simulink library "lib_all"  (Simulink 7.6)
settings: Solver=ode45  SolverMode=SingleTasking  StartTime=0.0  StopTime=10.0  RelTol=1e-3
values from neighbouring files (symbols this model uses):
  map_lib_03 = [6.8679 7.0159 7.4571 8.1849 9.1892 10.456 11.97 13.71 15.655 17.781 20.063 22.474 ... (803 elements, 73x11)]    [scr_simdata.mat]    (also in scr_simdata_all.mat)
  map_lib_06 = [2.5784 2.6727 2.9588 3.4453 4.1444 5.068 6.226 7.6242 9.2634 11.139 13.24 15.552 ... (803 elements, 73x11)]    [scr_simdata.mat]    (also in scr_simdata_all.mat)
  map_lib_09 = [0.0025784 0.0026727 0.0029588 0.0034453 0.0041444 0.005068 0.006226 0.0076242 0.0092634 0.011139 0.01324 0.015552 ... (803 elements, 73x11)]    [scr_simdata.mat]    (also in scr_simdata_all.mat)
  map_lib_10 = [2.74 2.75 2.76 2.77 2.78 2.79 2.8 2.81 2.82 2.83 2.84 2.85 ... (81 elements, 1x81)]    [scr_simdata.mat]    (also in scr_simdata_all.mat)
  map_lib_11 = [1.1983 1.2027 1.207 1.2113 1.2156 1.2198 1.2241 1.2283 1.2326 1.2368 1.241 1.2451 ... (81 elements, 1x81)]    [scr_simdata.mat]    (also in scr_simdata_all.mat)
  map_lib_12 = [2.74 2.75 2.76 2.77 2.78 2.79 2.8 2.81 2.82 2.83 2.84 2.85 ... (81 elements, 1x81)]    [scr_simdata.mat]    (also in scr_simdata_all.mat)
  map_lib_13 = [1.74 1.75 1.76 1.77 1.78 1.79 1.8 1.81 1.82 1.83 1.84 1.85 ... (81 elements, 1x81)]    [scr_simdata.mat]    (also in scr_simdata_all.mat)
  map_lib_16 = [0.00048828125 1.6159 1.8962 2.0821 2.2251 2.3426 2.4433 2.5318 2.611 2.683 2.749 2.8101 ... (1419 elements, 129x11)]    [scr_simdata.mat]    (also in scr_simdata_all.mat)
  map_mr2_01 = [0 800 1600 2400 3200 4000]    [scr_simdata.mat]    (also in scr_simdata_all.mat)
  map_mr2_02 = [1.32 1.32 1.33 1.34 1.36 1.38]    [scr_simdata.mat]    (also in scr_simdata_all.mat)
  map_mr2_03 = [0 800 1600 2400 3200 4000]    [scr_simdata.mat]    (also in scr_simdata_all.mat)
  map_mr2_04 = [1.32 1.32 1.33 1.34 1.36 1.38]    [scr_simdata.mat]    (also in scr_simdata_all.mat)
  map_mr2_05 = [0 800 1600 2400 3200 4000]    [scr_simdata.mat]    (also in scr_simdata_all.mat)
  map_mr2_06 = [1.32 1.32 1.33 1.34 1.36 1.38]    [scr_simdata.mat]    (also in scr_simdata_all.mat)
  Parameter1 = 6    [scr_simdata.mat]    (also in scr_simdata_all.mat)
  Parameter101 = 30    [scr_simdata.mat]    (also in scr_simdata_all.mat)
  Parameter102 = 12    [scr_simdata.mat]    (also in scr_simdata_all.mat)
  Parameter103 = -240    [scr_simdata.mat]    (also in scr_simdata_all.mat)
  Parameter108 = 48    [scr_simdata.mat]    (also in scr_simdata_all.mat)
  Parameter120 = 16    [scr_simdata.mat]    (also in scr_simdata_all.mat)
  Parameter121 = 87    [scr_simdata.mat]    (also in scr_simdata_all.mat)
  Parameter123 = 78    [scr_simdata.mat]    (also in scr_simdata_all.mat)
  Parameter124 = 60    [scr_simdata.mat]    (also in scr_simdata_all.mat)
  Parameter125 = -480    [scr_simdata.mat]    (also in scr_simdata_all.mat)
  Parameter130 = 96    [scr_simdata.mat]    (also in scr_simdata_all.mat)
  Parameter131 = 3    [scr_simdata.mat]    (also in scr_simdata_all.mat)
  Parameter144 = 3    [scr_simdata.mat]    (also in scr_simdata_all.mat)
  Parameter18 = 128    [scr_simdata.mat]    (also in scr_simdata_all.mat)
  Parameter19 = 16    [scr_simdata.mat]    (also in scr_simdata_all.mat)
  Parameter2 = 12    [scr_simdata.mat]    (also in scr_simdata_all.mat)
  Parameter20 = 7    [scr_simdata.mat]    (also in scr_simdata_all.mat)
  Parameter21 = 4    [scr_simdata.mat]    (also in scr_simdata_all.mat)
  Parameter3 = 18    [scr_simdata.mat]    (also in scr_simdata_all.mat)
  Parameter32 = 1    [scr_simdata.mat]    (also in scr_simdata_all.mat)
  Parameter34 = 24    [scr_simdata.mat]    (also in scr_simdata_all.mat)
  Parameter4 = 138    [scr_simdata.mat]    (also in scr_simdata_all.mat)
  Parameter42 = 48    [scr_simdata.mat]    (also in scr_simdata_all.mat)
  Parameter5 = 135    [scr_simdata.mat]    (also in scr_simdata_all.mat)
  Parameter55 = 0    [scr_simdata.mat]    (also in scr_simdata_all.mat)
  Parameter56 = 0    [scr_simdata.mat]    (also in scr_simdata_all.mat)
  Parameter57 = 1    [scr_simdata.mat]    (also in scr_simdata_all.mat)
  Parameter58 = 1    [scr_simdata.mat]    (also in scr_simdata_all.mat)
  Parameter59 = 2    [scr_simdata.mat]    (also in scr_simdata_all.mat)
  Parameter6 = 132    [scr_simdata.mat]    (also in scr_simdata_all.mat)
  Parameter60 = 2    [scr_simdata.mat]    (also in scr_simdata_all.mat)
  Parameter63 = 144    [scr_simdata.mat]    (also in scr_simdata_all.mat)
  Parameter7 = 126    [scr_simdata.mat]    (also in scr_simdata_all.mat)
  Parameter76 = 64    [scr_simdata.mat]    (also in scr_simdata_all.mat)
  Parameter77 = 135    [scr_simdata.mat]    (also in scr_simdata_all.mat)
  Parameter79 = 126    [scr_simdata.mat]    (also in scr_simdata_all.mat)
  Parameter80 = 108    [scr_simdata.mat]    (also in scr_simdata_all.mat)
  Parameter81 = 0    [scr_simdata.mat]    (also in scr_simdata_all.mat)
  Parameter86 = 0    [scr_simdata.mat]    (also in scr_simdata_all.mat)
  Parameter98 = 112    [scr_simdata.mat]    (also in scr_simdata_all.mat)
  Parameter99 = 39    [scr_simdata.mat]    (also in scr_simdata_all.mat)
undefined symbols (not defined in the file or in its folder): cylvolkp_hih_map2h, cylvolkp_map2h, pk1k_map2h
libraries: simulink x2
(part 20 of 36)

== system lib_all/SubSystem176/SubSystem329/SubSystem332/SubSystem337/SubSystem340 ==
  note: ***編集禁止***
  element : Inport
  Inside_DSR : DataStoreRead  DataStoreName=DSM379
  Selector : Selector  IndexOptions=Index vector (port)  Indices=[1 3]  OutputSizes=1
  Output : Outport
  wires:
  Selector/1 -> Output/1
  element/1 -> Selector/2
  Inside_DSR/1 -> Selector/1

== system lib_all/SubSystem176/SubSystem329/SubSystem332/SubSystem341/SubSystem342 ==
  note: ***編集禁止***
  input : Inport
  element : Inport  Port=2
  Assignment : Assignment  IndexOptions=Index vector (port)  Indices=1  OutputSizes=[1 1]
  Inside_DSR : DataStoreRead  DataStoreName=DSM378
  Inside_DSW : DataStoreWrite  DataStoreName=DSM378
  wires:
  element/1 -> Assignment/3
  input/1 -> Assignment/2
  Inside_DSR/1 -> Assignment/1
  Assignment/1 -> Inside_DSW/1

== system lib_all/SubSystem1770/SubSystem1774/SubSystem1778/SubSystem1779/SingleForIterator ==
  note: ***編集禁止***
  N : Inport
  For_loop : Stateflow chart  TreatAsAtomicUnit=on
  action : Outport
  i : Outport  Port=2
  wires:
  For_loop/2(function_call) -> action/1
  N/1 -> For_loop/1(N_Loop)
  For_loop/1(idx) -> i/1

== system lib_all/SubSystem1770/SubSystem1774/SubSystem1778/SubSystem1779/SubSystem1783 ==
  note: Text348
  Block4866 : Inport
  Block4867 : Inport  Port=2
  Block4868 : Inport  Port=3
  Block4869 : TriggerPort  TriggerType=function-call
  Block4870 : Constant  Value=0  OutDataTypeStr=double  SampleTime=-1
  Block4871 : Constant  Value=3  OutDataTypeStr=double  SampleTime=-1
  Block4872 : Constant  OutDataTypeStr=double  SampleTime=-1
  Block4873 : Constant  Value=Parameter63  OutDataTypeStr=double  SampleTime=-1
  Block4874 : Constant  OutDataTypeStr=double  SampleTime=-1
  Block4875 : Constant  OutDataTypeStr=double  SampleTime=-1
  Block4876 : Sum  Inputs=+-  OutDataTypeStr=double  RndMeth=Zero  SaturateOnIntegerOverflow=on  InputSameDT=on
  Block{4877..4881} (5 alike) : Sum  OutDataTypeStr=double  RndMeth=Zero  SaturateOnIntegerOverflow=on  InputSameDT=on
  Block4882 : Switch  Threshold=0.5  RndMeth=Zero  SaturateOnIntegerOverflow=on  InputSameDT=on
  Block4883 : Switch  Threshold=0.5  RndMeth=Zero  SaturateOnIntegerOverflow=on  InputSameDT=on
  Block4884 : UnitDelay
  SubSystem1784 : Subsystem
  SubSystem1785 : Subsystem
  SubSystem1786 : Subsystem
  Block4889 : Outport
  wires:
  Block4879/1 -> Block4882/3
  Block4884/1 -> Block4879/2
  SubSystem1786/1(Block4888) -> Block4879/1, Block4882/1
  Block4882/1 -> Block4884/1, Block4889/1
  Block4878/1 -> Block4883/1
  Block4873/1 -> Block4878/1
  SubSystem1784/1(Out1) -> Block4883/2
  SubSystem1785/1(Out1) -> Block4882/2
  Block4872/1 -> SubSystem1785/1(In1)
  Block4870/1 -> SubSystem1784/2(In2)
  Block4877/1 -> SubSystem1784/1(In1), Block4878/2, Block4883/3
  Block4880/1 -> SubSystem1786/1(Block4885)
  Block4867/1 -> Block4880/1
  Block4868/1 -> Block4877/2
  Block4876/1 -> Block4877/1
  Block4871/1 -> Block4876/2
  Block4866/1 -> SubSystem1785/2(In2), Block4876/1
  Block4874/1 -> Block4880/2
  Block4883/1 -> Block4881/1
  Block4875/1 -> Block4881/2
  Block4881/1 -> SubSystem1786/2(Block4886)

== system lib_all/SubSystem1770/SubSystem1774/SubSystem1791/SubSystem1792/SubSystem1794 ==
  note: ***編集禁止***
  N : Inport
  For_loop : Stateflow chart  TreatAsAtomicUnit=on
  action : Outport
  i : Outport  Port=2
  wires:
  For_loop/2(function_call) -> action/1
  N/1 -> For_loop/1(N_Loop)
  For_loop/1(idx) -> i/1

== system lib_all/SubSystem1770/SubSystem1774/SubSystem1791/SubSystem1792/SubSystem1796 ==
  note: Text351
  Block4905 : Inport
  Block4906 : Inport  Port=2
  Block4907 : Inport  Port=3
  Block4908 : TriggerPort  TriggerType=function-call
  Block4909 : Constant  Value=0  OutDataTypeStr=double  SampleTime=-1
  Block4910 : Constant  Value=3  OutDataTypeStr=double  SampleTime=-1
  Block4911 : Constant  OutDataTypeStr=double  SampleTime=-1
  Block4912 : Constant  Value=Parameter63  OutDataTypeStr=double  SampleTime=-1
  Block4913 : Constant  OutDataTypeStr=double  SampleTime=-1
  Block4914 : Constant  OutDataTypeStr=double  SampleTime=-1
  Block4915 : Sum  Inputs=+-  OutDataTypeStr=double  RndMeth=Zero  SaturateOnIntegerOverflow=on  InputSameDT=on
  Block{4916..4920} (5 alike) : Sum  OutDataTypeStr=double  RndMeth=Zero  SaturateOnIntegerOverflow=on  InputSameDT=on
  Block4921 : Switch  Threshold=0.5  RndMeth=Zero  SaturateOnIntegerOverflow=on  InputSameDT=on
  Block4922 : Switch  Threshold=0.5  RndMeth=Zero  SaturateOnIntegerOverflow=on  InputSameDT=on
  Block4923 : UnitDelay
  SubSystem1797 : Subsystem
  SubSystem1798 : Subsystem
  SubSystem1799 : Subsystem
  Block4928 : Outport
  wires:
  Block4918/1 -> Block4921/3
  Block4923/1 -> Block4918/2
  SubSystem1799/1(Block4927) -> Block4918/1, Block4921/1
  Block4921/1 -> Block4923/1, Block4928/1
  Block4917/1 -> Block4922/1
  Block4912/1 -> Block4917/1
  SubSystem1797/1(Out1) -> Block4922/2
  SubSystem1798/1(Out1) -> Block4921/2
  Block4911/1 -> SubSystem1798/1(In1)
  Block4909/1 -> SubSystem1797/2(In2)
  Block4916/1 -> SubSystem1797/1(In1), Block4917/2, Block4922/3
  Block4919/1 -> SubSystem1799/1(Block4924)
  Block4906/1 -> Block4919/1
  Block4907/1 -> Block4916/2
  Block4915/1 -> Block4916/1
  Block4910/1 -> Block4915/2
  Block4905/1 -> SubSystem1798/2(In2), Block4915/1
  Block4913/1 -> Block4919/2
  Block4922/1 -> Block4920/1
  Block4914/1 -> Block4920/2
  Block4920/1 -> SubSystem1799/2(Block4925)

== system lib_all/SubSystem1770/SubSystem1774/SubSystem1804/SubSystem1805/SubSystem1807 ==
  note: ***編集禁止***
  N : Inport
  For_loop : Stateflow chart  TreatAsAtomicUnit=on
  action : Outport
  i : Outport  Port=2
  wires:
  For_loop/2(function_call) -> action/1
  N/1 -> For_loop/1(N_Loop)
  For_loop/1(idx) -> i/1

== system lib_all/SubSystem1770/SubSystem1774/SubSystem1804/SubSystem1805/SubSystem1809 ==
  note: Text354
  Block4944 : Inport
  Block4945 : Inport  Port=2
  Block4946 : Inport  Port=3
  Block4947 : TriggerPort  TriggerType=function-call
  Block4948 : Constant  Value=0  OutDataTypeStr=double  SampleTime=-1
  Block4949 : Constant  Value=3  OutDataTypeStr=double  SampleTime=-1
  Block4950 : Constant  OutDataTypeStr=double  SampleTime=-1
  Block4951 : Constant  Value=Parameter63  OutDataTypeStr=double  SampleTime=-1
  Block4952 : Constant  OutDataTypeStr=double  SampleTime=-1
  Block4953 : Constant  OutDataTypeStr=double  SampleTime=-1
  Block4954 : Sum  Inputs=+-  OutDataTypeStr=double  RndMeth=Zero  SaturateOnIntegerOverflow=on  InputSameDT=on
  Block{4955..4959} (5 alike) : Sum  OutDataTypeStr=double  RndMeth=Zero  SaturateOnIntegerOverflow=on  InputSameDT=on
  Block4960 : Switch  Threshold=0.5  RndMeth=Zero  SaturateOnIntegerOverflow=on  InputSameDT=on
  Block4961 : Switch  Threshold=0.5  RndMeth=Zero  SaturateOnIntegerOverflow=on  InputSameDT=on
  Block4962 : UnitDelay
  SubSystem1810 : Subsystem
  SubSystem1811 : Subsystem
  SubSystem1812 : Subsystem
  Block4967 : Outport
  wires:
  Block4957/1 -> Block4960/3
  Block4962/1 -> Block4957/2
  SubSystem1812/1(Block4966) -> Block4957/1, Block4960/1
  Block4960/1 -> Block4962/1, Block4967/1
  Block4956/1 -> Block4961/1
  Block4951/1 -> Block4956/1
  SubSystem1810/1(Out1) -> Block4961/2
  SubSystem1811/1(Out1) -> Block4960/2
  Block4950/1 -> SubSystem1811/1(In1)
  Block4948/1 -> SubSystem1810/2(In2)
  Block4955/1 -> SubSystem1810/1(In1), Block4956/2, Block4961/3
  Block4958/1 -> SubSystem1812/1(Block4963)
  Block4945/1 -> Block4958/1
  Block4946/1 -> Block4955/2
  Block4954/1 -> Block4955/1
  Block4949/1 -> Block4954/2
  Block4944/1 -> SubSystem1811/2(In2), Block4954/1
  Block4952/1 -> Block4958/2
  Block4961/1 -> Block4959/1
  Block4953/1 -> Block4959/2
  Block4959/1 -> SubSystem1812/2(Block4964)

== system lib_all/SubSystem1770/SubSystem1817/SubSystem1821/SubSystem1822/SubSystem1824 ==
  note: ***編集禁止***
  N : Inport
  For_loop : Stateflow chart  TreatAsAtomicUnit=on
  action : Outport
  i : Outport  Port=2
  wires:
  For_loop/2(function_call) -> action/1
  N/1 -> For_loop/1(N_Loop)
  For_loop/1(idx) -> i/1

== system lib_all/SubSystem1770/SubSystem1817/SubSystem1821/SubSystem1822/SubSystem1826 ==
  note: Text357
  Block4992 : Inport
  Block4993 : Inport  Port=2
  Block4994 : Inport  Port=3
  Block4995 : TriggerPort  TriggerType=function-call
  Block4996 : Constant  Value=0  OutDataTypeStr=double  SampleTime=-1
  Block4997 : Constant  Value=3  OutDataTypeStr=double  SampleTime=-1
  Block4998 : Constant  OutDataTypeStr=double  SampleTime=-1
  Block4999 : Constant  Value=Parameter63  OutDataTypeStr=double  SampleTime=-1
  Block5000 : Constant  OutDataTypeStr=double  SampleTime=-1
  Block5001 : Constant  OutDataTypeStr=double  SampleTime=-1
  Block5002 : Sum  Inputs=+-  OutDataTypeStr=double  RndMeth=Zero  SaturateOnIntegerOverflow=on  InputSameDT=on
  Block{5003..5007} (5 alike) : Sum  OutDataTypeStr=double  RndMeth=Zero  SaturateOnIntegerOverflow=on  InputSameDT=on
  Block5008 : Switch  Threshold=0.5  RndMeth=Zero  SaturateOnIntegerOverflow=on  InputSameDT=on
  Block5009 : Switch  Threshold=0.5  RndMeth=Zero  SaturateOnIntegerOverflow=on  InputSameDT=on
  Block5010 : UnitDelay
  SubSystem1827 : Subsystem
  SubSystem1828 : Subsystem
  SubSystem1829 : Subsystem
  Block5015 : Outport
  wires:
  Block5005/1 -> Block5008/3
  Block5010/1 -> Block5005/2
  SubSystem1829/1(Block5014) -> Block5005/1, Block5008/1
  Block5008/1 -> Block5010/1, Block5015/1
  Block5004/1 -> Block5009/1
  Block4999/1 -> Block5004/1
  SubSystem1827/1(Out1) -> Block5009/2
  SubSystem1828/1(Out1) -> Block5008/2
  Block4998/1 -> SubSystem1828/1(In1)
  Block4996/1 -> SubSystem1827/2(In2)
  Block5003/1 -> SubSystem1827/1(In1), Block5004/2, Block5009/3
  Block5006/1 -> SubSystem1829/1(Block5011)
  Block4993/1 -> Block5006/1
  Block4994/1 -> Block5003/2
  Block5002/1 -> Block5003/1
  Block4997/1 -> Block5002/2
  Block4992/1 -> SubSystem1828/2(In2), Block5002/1
  Block5000/1 -> Block5006/2
  Block5009/1 -> Block5007/1
  Block5001/1 -> Block5007/2
  Block5007/1 -> SubSystem1829/2(Block5012)

== system lib_all/SubSystem1770/SubSystem1817/SubSystem1834/SubSystem1835/SubSystem1837 ==
  note: ***編集禁止***
  N : Inport
  For_loop : Stateflow chart  TreatAsAtomicUnit=on
  action : Outport
  i : Outport  Port=2
  wires:
  For_loop/2(function_call) -> action/1
  N/1 -> For_loop/1(N_Loop)
  For_loop/1(idx) -> i/1

== system lib_all/SubSystem1770/SubSystem1817/SubSystem1834/SubSystem1835/SubSystem1839 ==
  note: Text360
  Block5031 : Inport
  Block5032 : Inport  Port=2
  Block5033 : Inport  Port=3
  Block5034 : TriggerPort  TriggerType=function-call
  Block5035 : Constant  Value=0  OutDataTypeStr=double  SampleTime=-1
  Block5036 : Constant  Value=3  OutDataTypeStr=double  SampleTime=-1
  Block5037 : Constant  OutDataTypeStr=double  SampleTime=-1
  Block5038 : Constant  Value=Parameter63  OutDataTypeStr=double  SampleTime=-1
  Block5039 : Constant  OutDataTypeStr=double  SampleTime=-1
  Block5040 : Constant  OutDataTypeStr=double  SampleTime=-1
  Block5041 : Sum  Inputs=+-  OutDataTypeStr=double  RndMeth=Zero  SaturateOnIntegerOverflow=on  InputSameDT=on
  Block{5042..5046} (5 alike) : Sum  OutDataTypeStr=double  RndMeth=Zero  SaturateOnIntegerOverflow=on  InputSameDT=on
  Block5047 : Switch  Threshold=0.5  RndMeth=Zero  SaturateOnIntegerOverflow=on  InputSameDT=on
  Block5048 : Switch  Threshold=0.5  RndMeth=Zero  SaturateOnIntegerOverflow=on  InputSameDT=on
  Block5049 : UnitDelay
  SubSystem1840 : Subsystem
  SubSystem1841 : Subsystem
  SubSystem1842 : Subsystem
  Block5054 : Outport
  wires:
  Block5044/1 -> Block5047/3
  Block5049/1 -> Block5044/2
  SubSystem1842/1(Block5053) -> Block5044/1, Block5047/1
  Block5047/1 -> Block5049/1, Block5054/1
  Block5043/1 -> Block5048/1
  Block5038/1 -> Block5043/1
  SubSystem1840/1(Out1) -> Block5048/2
  SubSystem1841/1(Out1) -> Block5047/2
  Block5037/1 -> SubSystem1841/1(In1)
  Block5035/1 -> SubSystem1840/2(In2)
  Block5042/1 -> SubSystem1840/1(In1), Block5043/2, Block5048/3
  Block5045/1 -> SubSystem1842/1(Block5050)
  Block5032/1 -> Block5045/1
  Block5033/1 -> Block5042/2
  Block5041/1 -> Block5042/1
  Block5036/1 -> Block5041/2
  Block5031/1 -> SubSystem1841/2(In2), Block5041/1
  Block5039/1 -> Block5045/2
  Block5048/1 -> Block5046/1
  Block5040/1 -> Block5046/2
  Block5046/1 -> SubSystem1842/2(Block5051)

== system lib_all/SubSystem1770/SubSystem1817/SubSystem1847/SubSystem1848/SubSystem1850 ==
  note: ***編集禁止***
  N : Inport
  For_loop : Stateflow chart  TreatAsAtomicUnit=on
  action : Outport
  i : Outport  Port=2
  wires:
  For_loop/2(function_call) -> action/1
  N/1 -> For_loop/1(N_Loop)
  For_loop/1(idx) -> i/1

== system lib_all/SubSystem1770/SubSystem1817/SubSystem1847/SubSystem1848/SubSystem1852 ==
  note: Text363
  Block5070 : Inport
  Block5071 : Inport  Port=2
  Block5072 : Inport  Port=3
  Block5073 : TriggerPort  TriggerType=function-call
  Block5074 : Constant  Value=0  OutDataTypeStr=double  SampleTime=-1
  Block5075 : Constant  Value=3  OutDataTypeStr=double  SampleTime=-1
  Block5076 : Constant  OutDataTypeStr=double  SampleTime=-1
  Block5077 : Constant  Value=Parameter63  OutDataTypeStr=double  SampleTime=-1
  Block5078 : Constant  OutDataTypeStr=double  SampleTime=-1
  Block5079 : Constant  OutDataTypeStr=double  SampleTime=-1
  Block5080 : Sum  Inputs=+-  OutDataTypeStr=double  RndMeth=Zero  SaturateOnIntegerOverflow=on  InputSameDT=on
  Block{5081..5085} (5 alike) : Sum  OutDataTypeStr=double  RndMeth=Zero  SaturateOnIntegerOverflow=on  InputSameDT=on
  Block5086 : Switch  Threshold=0.5  RndMeth=Zero  SaturateOnIntegerOverflow=on  InputSameDT=on
  Block5087 : Switch  Threshold=0.5  RndMeth=Zero  SaturateOnIntegerOverflow=on  InputSameDT=on
  Block5088 : UnitDelay
  SubSystem1853 : Subsystem
  SubSystem1854 : Subsystem
  SubSystem1855 : Subsystem
  Block5093 : Outport
  wires:
  Block5083/1 -> Block5086/3
  Block5088/1 -> Block5083/2
  SubSystem1855/1(Block5092) -> Block5083/1, Block5086/1
  Block5086/1 -> Block5088/1, Block5093/1
  Block5082/1 -> Block5087/1
  Block5077/1 -> Block5082/1
  SubSystem1853/1(Out1) -> Block5087/2
  SubSystem1854/1(Out1) -> Block5086/2
  Block5076/1 -> SubSystem1854/1(In1)
  Block5074/1 -> SubSystem1853/2(In2)
  Block5081/1 -> SubSystem1853/1(In1), Block5082/2, Block5087/3
  Block5084/1 -> SubSystem1855/1(Block5089)
  Block5071/1 -> Block5084/1
  Block5072/1 -> Block5081/2
  Block5080/1 -> Block5081/1
  Block5075/1 -> Block5080/2
  Block5070/1 -> SubSystem1854/2(In2), Block5080/1
  Block5078/1 -> Block5084/2
  Block5087/1 -> Block5085/1
  Block5079/1 -> Block5085/2
  Block5085/1 -> SubSystem1855/2(Block5090)

== system lib_all/SubSystem1879/SubSystem1887/SubSystem1891/SubSystem1892/SubSystem1893 ==
  note: ***編集禁止***
  element : Inport
  Inside_DSR : DataStoreRead  DataStoreName=DSM18
  Selector : Selector  IndexOptions=Index vector (port)  Indices=[1 3]  OutputSizes=1
  Output : Outport
  wires:
  Inside_DSR/1 -> Selector/1
  element/1 -> Selector/2
  Selector/1 -> Output/1

== system lib_all/SubSystem1879/SubSystem1887/SubSystem1891/SubSystem1892/SubSystem1894 ==
  note: ***編集禁止***
  element : Inport
  Inside_DSR : DataStoreRead  DataStoreName=DSM8
  Selector : Selector  IndexOptions=Index vector (port)  Indices=[1 3]  OutputSizes=1
  Output : Outport
  wires:
  Selector/1 -> Output/1
  element/1 -> Selector/2
  Inside_DSR/1 -> Selector/1

== system lib_all/SubSystem1879/SubSystem1887/SubSystem1891/SubSystem1892/SubSystem1895 ==
  note: ***編集禁止***
  element : Inport
  Inside_DSR : DataStoreRead  DataStoreName=DSM11
  Selector : Selector  IndexOptions=Index vector (port)  Indices=[1 3]  OutputSizes=1
  Output : Outport
  wires:
  Inside_DSR/1 -> Selector/1
  element/1 -> Selector/2
  Selector/1 -> Output/1

== system lib_all/SubSystem1879/SubSystem1887/SubSystem1891/SubSystem1896/SubSystem1897 ==
  note: ***編集禁止***
  element : Inport
  Inside_DSR : DataStoreRead  DataStoreName=DSM18
  Selector : Selector  IndexOptions=Index vector (port)  Indices=[1 3]  OutputSizes=1
  Output : Outport
  wires:
  Inside_DSR/1 -> Selector/1
  element/1 -> Selector/2
  Selector/1 -> Output/1

== system lib_all/SubSystem1879/SubSystem1887/SubSystem1891/SubSystem1896/SubSystem1898 ==
  note: ***編集禁止***
  element : Inport
  Inside_DSR : DataStoreRead  DataStoreName=DSM8
  Selector : Selector  IndexOptions=Index vector (port)  Indices=[1 3]  OutputSizes=1
  Output : Outport
  wires:
  Selector/1 -> Output/1
  element/1 -> Selector/2
  Inside_DSR/1 -> Selector/1

== system lib_all/SubSystem1879/SubSystem1887/SubSystem1891/SubSystem1896/SubSystem1899 ==
  note: ***編集禁止***
  element : Inport
  Inside_DSR : DataStoreRead  DataStoreName=DSM11
  Selector : Selector  IndexOptions=Index vector (port)  Indices=[1 3]  OutputSizes=1
  Output : Outport
  wires:
  Inside_DSR/1 -> Selector/1
  element/1 -> Selector/2
  Selector/1 -> Output/1

== system lib_all/SubSystem1879/SubSystem1887/SubSystem1900/SubSystem1902/SubSystem1903 ==
  note: ***編集禁止***
  element : Inport
  Inside_DSR : DataStoreRead  DataStoreName=DSM18
  Selector : Selector  IndexOptions=Index vector (port)  Indices=[1 3]  OutputSizes=1
  Output : Outport
  wires:
  Inside_DSR/1 -> Selector/1
  element/1 -> Selector/2
  Selector/1 -> Output/1

== system lib_all/SubSystem1879/SubSystem1887/SubSystem1900/SubSystem1902/SubSystem1904 ==
  note: ***編集禁止***
  element : Inport
  Inside_DSR : DataStoreRead  DataStoreName=DSM8
  Selector : Selector  IndexOptions=Index vector (port)  Indices=[1 3]  OutputSizes=1
  Output : Outport
  wires:
  Selector/1 -> Output/1
  element/1 -> Selector/2
  Inside_DSR/1 -> Selector/1

== system lib_all/SubSystem1879/SubSystem1887/SubSystem1900/SubSystem1902/SubSystem1905 ==
  note: ***編集禁止***
  element : Inport
  Inside_DSR : DataStoreRead  DataStoreName=DSM11
  Selector : Selector  IndexOptions=Index vector (port)  Indices=[1 3]  OutputSizes=1
  Output : Outport
  wires:
  Inside_DSR/1 -> Selector/1
  element/1 -> Selector/2
  Selector/1 -> Output/1

== system lib_all/SubSystem1879/SubSystem1887/SubSystem1900/SubSystem1906/SubSystem1907 ==
  note: ***編集禁止***
  element : Inport
  Inside_DSR : DataStoreRead  DataStoreName=DSM18
  Selector : Selector  IndexOptions=Index vector (port)  Indices=[1 3]  OutputSizes=1
  Output : Outport
  wires:
  Inside_DSR/1 -> Selector/1
  element/1 -> Selector/2
  Selector/1 -> Output/1

== system lib_all/SubSystem1879/SubSystem1887/SubSystem1900/SubSystem1906/SubSystem1908 ==
  note: ***編集禁止***
  element : Inport
  Inside_DSR : DataStoreRead  DataStoreName=DSM8
  Selector : Selector  IndexOptions=Index vector (port)  Indices=[1 3]  OutputSizes=1
  Output : Outport
  wires:
  Selector/1 -> Output/1
  element/1 -> Selector/2
  Inside_DSR/1 -> Selector/1

== system lib_all/SubSystem1879/SubSystem1887/SubSystem1900/SubSystem1906/SubSystem1909 ==
  note: ***編集禁止***
  element : Inport
  Inside_DSR : DataStoreRead  DataStoreName=DSM11
  Selector : Selector  IndexOptions=Index vector (port)  Indices=[1 3]  OutputSizes=1
  Output : Outport
  wires:
  Inside_DSR/1 -> Selector/1
  element/1 -> Selector/2
  Selector/1 -> Output/1

== system lib_all/SubSystem1879/SubSystem1887/SubSystem1910/SubSystem1912/SubSystem1913 ==
  note: ***編集禁止***
  element : Inport
  Inside_DSR : DataStoreRead  DataStoreName=DSM18
  Selector : Selector  IndexOptions=Index vector (port)  Indices=[1 3]  OutputSizes=1
  Output : Outport
  wires:
  Inside_DSR/1 -> Selector/1
  element/1 -> Selector/2
  Selector/1 -> Output/1

== system lib_all/SubSystem1879/SubSystem1887/SubSystem1910/SubSystem1912/SubSystem1914 ==
  note: ***編集禁止***
  element : Inport
  Inside_DSR : DataStoreRead  DataStoreName=DSM8
  Selector : Selector  IndexOptions=Index vector (port)  Indices=[1 3]  OutputSizes=1
  Output : Outport
  wires:
  Selector/1 -> Output/1
  element/1 -> Selector/2
  Inside_DSR/1 -> Selector/1

== system lib_all/SubSystem1879/SubSystem1887/SubSystem1910/SubSystem1912/SubSystem1915 ==
  note: ***編集禁止***
  element : Inport
  Inside_DSR : DataStoreRead  DataStoreName=DSM11
  Selector : Selector  IndexOptions=Index vector (port)  Indices=[1 3]  OutputSizes=1
  Output : Outport
  wires:
  Inside_DSR/1 -> Selector/1
  element/1 -> Selector/2
  Selector/1 -> Output/1

== system lib_all/SubSystem1879/SubSystem1887/SubSystem1910/SubSystem1916/SubSystem1917 ==
  note: ***編集禁止***
  element : Inport
  Inside_DSR : DataStoreRead  DataStoreName=DSM18
  Selector : Selector  IndexOptions=Index vector (port)  Indices=[1 3]  OutputSizes=1
  Output : Outport
  wires:
  Inside_DSR/1 -> Selector/1
  element/1 -> Selector/2
  Selector/1 -> Output/1

== system lib_all/SubSystem1879/SubSystem1887/SubSystem1910/SubSystem1916/SubSystem1918 ==
  note: ***編集禁止***
  element : Inport
  Inside_DSR : DataStoreRead  DataStoreName=DSM8
  Selector : Selector  IndexOptions=Index vector (port)  Indices=[1 3]  OutputSizes=1
  Output : Outport
  wires:
  Selector/1 -> Output/1
  element/1 -> Selector/2
  Inside_DSR/1 -> Selector/1

== system lib_all/SubSystem1879/SubSystem1887/SubSystem1910/SubSystem1916/SubSystem1919 ==
  note: ***編集禁止***
  element : Inport
  Inside_DSR : DataStoreRead  DataStoreName=DSM11
  Selector : Selector  IndexOptions=Index vector (port)  Indices=[1 3]  OutputSizes=1
  Output : Outport
  wires:
  Inside_DSR/1 -> Selector/1
  element/1 -> Selector/2
  Selector/1 -> Output/1

== system lib_all/SubSystem1920/SubSystem1928/SubSystem1932/SubSystem1933/SubSystem1934 ==
  note: ***編集禁止***
  element : Inport
  Inside_DSR : DataStoreRead  DataStoreName=DSM18
  Selector : Selector  IndexOptions=Index vector (port)  Indices=[1 3]  OutputSizes=1
  Output : Outport
  wires:
  Inside_DSR/1 -> Selector/1
  element/1 -> Selector/2
  Selector/1 -> Output/1

== system lib_all/SubSystem1920/SubSystem1928/SubSystem1932/SubSystem1933/SubSystem1935 ==
  note: ***編集禁止***
  element : Inport
  Inside_DSR : DataStoreRead  DataStoreName=DSM8
  Selector : Selector  IndexOptions=Index vector (port)  Indices=[1 3]  OutputSizes=1
  Output : Outport
  wires:
  Selector/1 -> Output/1
  element/1 -> Selector/2
  Inside_DSR/1 -> Selector/1

== system lib_all/SubSystem1920/SubSystem1928/SubSystem1932/SubSystem1933/SubSystem1936 ==
  note: ***編集禁止***
  element : Inport
  Inside_DSR : DataStoreRead  DataStoreName=DSM11
  Selector : Selector  IndexOptions=Index vector (port)  Indices=[1 3]  OutputSizes=1
  Output : Outport
  wires:
  Inside_DSR/1 -> Selector/1
  element/1 -> Selector/2
  Selector/1 -> Output/1

== system lib_all/SubSystem1920/SubSystem1928/SubSystem1932/SubSystem1937/SubSystem1938 ==
  note: ***編集禁止***
  element : Inport
  Inside_DSR : DataStoreRead  DataStoreName=DSM18
  Selector : Selector  IndexOptions=Index vector (port)  Indices=[1 3]  OutputSizes=1
  Output : Outport
  wires:
  Inside_DSR/1 -> Selector/1
  element/1 -> Selector/2
  Selector/1 -> Output/1

== system lib_all/SubSystem1920/SubSystem1928/SubSystem1932/SubSystem1937/SubSystem1939 ==
  note: ***編集禁止***
  element : Inport
  Inside_DSR : DataStoreRead  DataStoreName=DSM8
  Selector : Selector  IndexOptions=Index vector (port)  Indices=[1 3]  OutputSizes=1
  Output : Outport
  wires:
  Selector/1 -> Output/1
  element/1 -> Selector/2
  Inside_DSR/1 -> Selector/1

== system lib_all/SubSystem1920/SubSystem1928/SubSystem1932/SubSystem1937/SubSystem1940 ==
  note: ***編集禁止***
  element : Inport
  Inside_DSR : DataStoreRead  DataStoreName=DSM11
  Selector : Selector  IndexOptions=Index vector (port)  Indices=[1 3]  OutputSizes=1
  Output : Outport
  wires:
  Inside_DSR/1 -> Selector/1
  element/1 -> Selector/2
  Selector/1 -> Output/1

== system lib_all/SubSystem1920/SubSystem1928/SubSystem1941/SubSystem1943/SubSystem1944 ==
  note: ***編集禁止***
  element : Inport
  Inside_DSR : DataStoreRead  DataStoreName=DSM18
  Selector : Selector  IndexOptions=Index vector (port)  Indices=[1 3]  OutputSizes=1
  Output : Outport
  wires:
  Inside_DSR/1 -> Selector/1
  element/1 -> Selector/2
  Selector/1 -> Output/1

== system lib_all/SubSystem1920/SubSystem1928/SubSystem1941/SubSystem1943/SubSystem1945 ==
  note: ***編集禁止***
  element : Inport
  Inside_DSR : DataStoreRead  DataStoreName=DSM8
  Selector : Selector  IndexOptions=Index vector (port)  Indices=[1 3]  OutputSizes=1
  Output : Outport
  wires:
  Selector/1 -> Output/1
  element/1 -> Selector/2
  Inside_DSR/1 -> Selector/1

== system lib_all/SubSystem1920/SubSystem1928/SubSystem1941/SubSystem1943/SubSystem1946 ==
  note: ***編集禁止***
  element : Inport
  Inside_DSR : DataStoreRead  DataStoreName=DSM11
  Selector : Selector  IndexOptions=Index vector (port)  Indices=[1 3]  OutputSizes=1
  Output : Outport
  wires:
  Inside_DSR/1 -> Selector/1
  element/1 -> Selector/2
  Selector/1 -> Output/1

== system lib_all/SubSystem1920/SubSystem1928/SubSystem1941/SubSystem1947/SubSystem1948 ==
  note: ***編集禁止***
  element : Inport
  Inside_DSR : DataStoreRead  DataStoreName=DSM18
  Selector : Selector  IndexOptions=Index vector (port)  Indices=[1 3]  OutputSizes=1
  Output : Outport
  wires:
  Inside_DSR/1 -> Selector/1
  element/1 -> Selector/2
  Selector/1 -> Output/1

== system lib_all/SubSystem1920/SubSystem1928/SubSystem1941/SubSystem1947/SubSystem1949 ==
  note: ***編集禁止***
  element : Inport
  Inside_DSR : DataStoreRead  DataStoreName=DSM8
  Selector : Selector  IndexOptions=Index vector (port)  Indices=[1 3]  OutputSizes=1
  Output : Outport
  wires:
  Selector/1 -> Output/1
  element/1 -> Selector/2
  Inside_DSR/1 -> Selector/1

== system lib_all/SubSystem1920/SubSystem1928/SubSystem1941/SubSystem1947/SubSystem1950 ==
  note: ***編集禁止***
  element : Inport
  Inside_DSR : DataStoreRead  DataStoreName=DSM11
  Selector : Selector  IndexOptions=Index vector (port)  Indices=[1 3]  OutputSizes=1
  Output : Outport
  wires:
  Inside_DSR/1 -> Selector/1
  element/1 -> Selector/2
  Selector/1 -> Output/1

== system lib_all/SubSystem1920/SubSystem1928/SubSystem1951/SubSystem1953/SubSystem1954 ==
  note: ***編集禁止***
  element : Inport
  Inside_DSR : DataStoreRead  DataStoreName=DSM18
  Selector : Selector  IndexOptions=Index vector (port)  Indices=[1 3]  OutputSizes=1
  Output : Outport
  wires:
  Inside_DSR/1 -> Selector/1
  element/1 -> Selector/2
  Selector/1 -> Output/1

== system lib_all/SubSystem1920/SubSystem1928/SubSystem1951/SubSystem1953/SubSystem1955 ==
  note: ***編集禁止***
  element : Inport
  Inside_DSR : DataStoreRead  DataStoreName=DSM8
  Selector : Selector  IndexOptions=Index vector (port)  Indices=[1 3]  OutputSizes=1
  Output : Outport
  wires:
  Selector/1 -> Output/1
  element/1 -> Selector/2
  Inside_DSR/1 -> Selector/1

== system lib_all/SubSystem1920/SubSystem1928/SubSystem1951/SubSystem1953/SubSystem1956 ==
  note: ***編集禁止***
  element : Inport
  Inside_DSR : DataStoreRead  DataStoreName=DSM11
  Selector : Selector  IndexOptions=Index vector (port)  Indices=[1 3]  OutputSizes=1
  Output : Outport
  wires:
  Inside_DSR/1 -> Selector/1
  element/1 -> Selector/2
  Selector/1 -> Output/1

== system lib_all/SubSystem1920/SubSystem1928/SubSystem1951/SubSystem1957/SubSystem1958 ==
  note: ***編集禁止***
  element : Inport
  Inside_DSR : DataStoreRead  DataStoreName=DSM18
  Selector : Selector  IndexOptions=Index vector (port)  Indices=[1 3]  OutputSizes=1
  Output : Outport
  wires:
  Inside_DSR/1 -> Selector/1
  element/1 -> Selector/2
  Selector/1 -> Output/1

== system lib_all/SubSystem1920/SubSystem1928/SubSystem1951/SubSystem1957/SubSystem1959 ==
  note: ***編集禁止***
  element : Inport
  Inside_DSR : DataStoreRead  DataStoreName=DSM8
  Selector : Selector  IndexOptions=Index vector (port)  Indices=[1 3]  OutputSizes=1
  Output : Outport
  wires:
  Selector/1 -> Output/1
  element/1 -> Selector/2
  Inside_DSR/1 -> Selector/1

== system lib_all/SubSystem1920/SubSystem1928/SubSystem1951/SubSystem1957/SubSystem1960 ==
  note: ***編集禁止***
  element : Inport
  Inside_DSR : DataStoreRead  DataStoreName=DSM11
  Selector : Selector  IndexOptions=Index vector (port)  Indices=[1 3]  OutputSizes=1
  Output : Outport
  wires:
  Inside_DSR/1 -> Selector/1
  element/1 -> Selector/2
  Selector/1 -> Output/1

== system lib_all/SubSystem347/SubSystem349/SubSystem352/SubSystem353/SubSystem355 ==
  note: ***編集禁止***
  In1 : Inport
  In2 : Inport  Port=2
  LO1 : Logic
  RO1 : RelationalOperator  Operator=<  InputSameDT=on
  RO2 : RelationalOperator  Operator=~=  InputSameDT=on
  single1 : DataTypeConversion  OutDataTypeStr=single  SaturateOnIntegerOverflow=on  RndMeth=Zero
  single2 : DataTypeConversion  OutDataTypeStr=single  SaturateOnIntegerOverflow=on  RndMeth=Zero
  Out1 : Outport
  wires:
  In2/1 -> single2/1
  In1/1 -> single1/1
  single1/1 -> RO1/1, RO2/1
  single2/1 -> RO1/2, RO2/2
  RO1/1 -> LO1/1
  RO2/1 -> LO1/2
  LO1/1 -> Out1/1

== system lib_all/SubSystem347/SubSystem349/SubSystem352/SubSystem353/SubSystem356 ==
  note: Text54
  note: Text53
  Block789 : Inport
  Block790 : Inport  Port=2
  Block791 : Inport  Port=3
  Block792 : Constant  Value=720  OutDataTypeStr=double  SampleTime=-1
  Block793 : Constant  Value=0  OutDataTypeStr=double  SampleTime=-1
  Block794 : Constant  Value=0  OutDataTypeStr=double  SampleTime=-1
  Block795 : Constant  Value=5  OutDataTypeStr=double  SampleTime=-1
  Block796 : DataStoreRead  DataStoreName=DSM50
  Block797 : DataStoreRead  DataStoreName=DSM35
  Block798 : Gain  Gain=5  SaturateOnIntegerOverflow=on  OutDataTypeStr=Inherit: Same as input  ParamDataTypeStr=Inherit: Same as input
      settings: AttributesFormatString=Attribute180
  Block799 : Gain  Gain=5  SaturateOnIntegerOverflow=on  OutDataTypeStr=Inherit: Same as input  ParamDataTypeStr=Inherit: Same as input
      settings: AttributesFormatString=Attribute181
  Block800 : Gain  Gain=1/5  SaturateOnIntegerOverflow=on  OutDataTypeStr=Inherit: Same as input  ParamDataTypeStr=Inherit: Same as input
      settings: AttributesFormatString=Attribute182
  Block801 : Product  Inputs=*/  OutDataTypeStr=double  SaturateOnIntegerOverflow=on  RndMeth=Zero  InputSameDT=on
  Block802 : Rounding
  Block803 : Rounding
  Block804 : Sum  Inputs=+-+-  OutDataTypeStr=double  RndMeth=Zero  SaturateOnIntegerOverflow=on  InputSameDT=on
  Block805 : Sum  Inputs=-+  OutDataTypeStr=double  RndMeth=Zero  SaturateOnIntegerOverflow=on  InputSameDT=on
  SubSystem357 : Subsystem  TreatAsAtomicUnit=on  mask(***ATC.DIVISION***){bd_version=1.01.A, r0=チェック有り, r1=, r2=, r3=, r4=, r5=, r6=, r7=, r8=, r9=, c0=, c1=, c2=, c3=, c4=, c5=, c6=, c7=, c8=, c9=}
      mask prompts: bd_version "Version"
      mask help: これはAutoCからのブロックです．編集禁止．
      settings: OpenFcn=warndlg('変更禁止!');
      mask Help: web(ui_blockhelpmng(gcb));
  SubSystem358 : Subsystem  TreatAsAtomicUnit=on  mask(***ATC.DSRVEC***){bd_version=1.01.A, r0=DSM95, r1=-1, r2=, r3=, r4=, r5=, r6=, r7=, r8=, r9=, c0=, c1=, c2=, c3=, c4=, c5=, c6=, c7=, c8=, c9=}
      mask prompts: bd_version "Version"
      mask help: これはAutoCからのブロックです．編集禁止．
      settings: OpenFcn=warndlg('変更禁止!');
      mask Help: web(ui_blockhelpmng(gcb));
      mask Display: disp('ATC.DSRVEC')
  SubSystem359 : Subsystem
      settings: RTWSystemCode=Inline
  Block814 : Outport
  Block815 : Outport  Port=2
  wires:
  Block803/1 -> Block814/1
  Block800/1 -> Block803/1
  Block799/1 -> Block804/1
  Block796/1 -> Block804/2
  SubSystem358/1(Output) -> SubSystem357/1(mult)
  Block793/1 -> SubSystem357/3(init)
  SubSystem357/1(Out) -> Block804/3  "SigLabel18"
  Block789/1 -> Block799/1
  SubSystem359/1(Block813) -> Block800/1, Block805/2, Block801/1
  Block791/1 -> Block804/4
  Block792/1 -> SubSystem359/1(Block806)
  Block804/1 -> SubSystem359/2(Block807)  "SigLabel17"
  Block794/1 -> SubSystem359/3(Block808)
  Block790/1 -> SubSystem358/1(element)
  Block795/1 -> Block801/2
  Block801/1 -> Block802/1
  Block802/1 -> Block798/1
  Block798/1 -> Block805/1
  Block805/1 -> Block815/1
  Block797/1 -> SubSystem357/2(div)

== system lib_all/SubSystem347/SubSystem349/SubSystem352/SubSystem353/SubSystem362 ==
  note: Text57
  note: Text56
  note: Text55
  Block816 : Inport
  Block817 : Inport  Port=2
  Block818 : Inport  Port=3
  Block819 : Constant  Value=0  OutDataTypeStr=double  SampleTime=-1
  Block820 : Constant  OutDataTypeStr=double  SampleTime=-1
  Block821 : Constant  OutDataTypeStr=double  SampleTime=-1
  Block822 : Constant  Value=0  OutDataTypeStr=double  SampleTime=-1
  Block823 : Constant  Value=2  OutDataTypeStr=double  SampleTime=-1
  Block824 : Constant  OutDataTypeStr=double  SampleTime=-1
  Block825 : Constant  Value=143  OutDataTypeStr=double  SampleTime=-1
  Block826 : Demux  Outputs=2
  Block827 : Logic  Operator=NOT
  Block828 : Mux  Inputs=2
  Block829 : Mux  Inputs=2
  Block830 : Sum  OutDataTypeStr=double  RndMeth=Zero  SaturateOnIntegerOverflow=on  InputSameDT=on
  Block831 : Sum  OutDataTypeStr=double  RndMeth=Zero  SaturateOnIntegerOverflow=on  InputSameDT=on
  Block{832..835} (4 alike) : Switch  Threshold=0.5  RndMeth=Zero  SaturateOnIntegerOverflow=on  InputSameDT=on
  SubSystem363 : Subsystem
  SubSystem364 : Subsystem
  SubSystem365 : Subsystem
  SubSystem366 : Subsystem
  SubSystem367 : Subsystem  TreatAsAtomicUnit=on
  SubSystem376 : Subsystem  TreatAsAtomicUnit=on
  SubSystem386 : Subsystem  TreatAsAtomicUnit=on
  SubSystem396 : Subsystem  TreatAsAtomicUnit=on
  Block895 : Outport
  Block896 : Outport  Port=2
  wires:
  Block834/1 -> Block831/2
  Block824/1 -> Block831/1
  Block816/1 -> SubSystem363/1(In1), Block830/2, Block828/1
  Block825/1 -> SubSystem363/2(In2), Block829/1
  Block817/1 -> SubSystem364/1(In1), SubSystem365/1(In1), SubSystem366/1(In1)
  Block819/1 -> SubSystem364/2(In2)
  SubSystem364/1(Out1) -> Block832/2, SubSystem367/Enable
  SubSystem367/1(Block849) -> Block832/1
  Block820/1 -> SubSystem365/2(In2)
  SubSystem365/1(Out1) -> Block833/2, SubSystem376/Enable
  SubSystem376/1(Block864) -> Block833/1
  Block833/1 -> Block832/3
  Block831/1 -> SubSystem367/1(Block836), SubSystem376/1(Block850), SubSystem386/1(Block865), SubSystem396/1(Block880)
  Block826/1 -> Block895/1
  Block826/2 -> Block896/1
  Block832/1 -> Block826/1
  SubSystem363/1(Out1) -> Block834/2
  Block821/1 -> Block830/1
  Block822/1 -> Block829/2
  Block829/1 -> Block834/3
  Block830/1 -> Block828/2
  Block828/1 -> Block834/1
  Block823/1 -> SubSystem366/2(In2)
  SubSystem366/1(Out1) -> Block827/1, SubSystem386/Enable, Block835/2
  Block827/1 -> SubSystem396/Enable
  SubSystem386/1(Block879) -> Block835/1
  SubSystem396/1(Block894) -> Block835/3
  Block835/1 -> Block833/3
  Block818/1 -> SubSystem367/2(Block837), SubSystem376/2(Block851), SubSystem386/2(Block866), SubSystem396/2(Block881)

== system lib_all/SubSystem347/SubSystem349/SubSystem352/SubSystem406/SubSystem408 ==
  note: ***編集禁止***
  In1 : Inport
  In2 : Inport  Port=2
  LO1 : Logic
  RO1 : RelationalOperator  Operator=<  InputSameDT=on
  RO2 : RelationalOperator  Operator=~=  InputSameDT=on
  single1 : DataTypeConversion  OutDataTypeStr=single  SaturateOnIntegerOverflow=on  RndMeth=Zero
  single2 : DataTypeConversion  OutDataTypeStr=single  SaturateOnIntegerOverflow=on  RndMeth=Zero
  Out1 : Outport
  wires:
  In2/1 -> single2/1
  In1/1 -> single1/1
  single1/1 -> RO1/1, RO2/1
  single2/1 -> RO1/2, RO2/2
  RO1/1 -> LO1/1
  RO2/1 -> LO1/2
  LO1/1 -> Out1/1

== system lib_all/SubSystem347/SubSystem349/SubSystem352/SubSystem406/SubSystem409 ==
  note: Text60
  note: Text59
  Block911 : Inport
  Block912 : Inport  Port=2
  Block913 : Inport  Port=3
  Block914 : Constant  Value=720  OutDataTypeStr=double  SampleTime=-1
  Block915 : Constant  Value=0  OutDataTypeStr=double  SampleTime=-1
  Block916 : Constant  Value=0  OutDataTypeStr=double  SampleTime=-1
  Block917 : Constant  Value=5  OutDataTypeStr=double  SampleTime=-1
  Block918 : DataStoreRead  DataStoreName=DSM50
  Block919 : DataStoreRead  DataStoreName=DSM35
  Block920 : Gain  Gain=5  SaturateOnIntegerOverflow=on  OutDataTypeStr=Inherit: Same as input  ParamDataTypeStr=Inherit: Same as input
      settings: AttributesFormatString=Attribute199
  Block921 : Gain  Gain=5  SaturateOnIntegerOverflow=on  OutDataTypeStr=Inherit: Same as input  ParamDataTypeStr=Inherit: Same as input
      settings: AttributesFormatString=Attribute200
  Block922 : Gain  Gain=1/5  SaturateOnIntegerOverflow=on  OutDataTypeStr=Inherit: Same as input  ParamDataTypeStr=Inherit: Same as input
      settings: AttributesFormatString=Attribute201
  Block923 : Product  Inputs=*/  OutDataTypeStr=double  SaturateOnIntegerOverflow=on  RndMeth=Zero  InputSameDT=on
  Block924 : Rounding
  Block925 : Rounding
  Block926 : Sum  Inputs=+-+-  OutDataTypeStr=double  RndMeth=Zero  SaturateOnIntegerOverflow=on  InputSameDT=on
  Block927 : Sum  Inputs=-+  OutDataTypeStr=double  RndMeth=Zero  SaturateOnIntegerOverflow=on  InputSameDT=on
  SubSystem410 : Subsystem  TreatAsAtomicUnit=on  mask(***ATC.DIVISION***){bd_version=1.01.A, r0=チェック有り, r1=, r2=, r3=, r4=, r5=, r6=, r7=, r8=, r9=, c0=, c1=, c2=, c3=, c4=, c5=, c6=, c7=, c8=, c9=}
      mask prompts: bd_version "Version"
      mask help: これはAutoCからのブロックです．編集禁止．
      settings: OpenFcn=warndlg('変更禁止!');
      mask Help: web(ui_blockhelpmng(gcb));
  SubSystem411 : Subsystem  TreatAsAtomicUnit=on  mask(***ATC.DSRVEC***){bd_version=1.01.A, r0=DSM95, r1=-1, r2=, r3=, r4=, r5=, r6=, r7=, r8=, r9=, c0=, c1=, c2=, c3=, c4=, c5=, c6=, c7=, c8=, c9=}
      mask prompts: bd_version "Version"
      mask help: これはAutoCからのブロックです．編集禁止．
      settings: OpenFcn=warndlg('変更禁止!');
      mask Help: web(ui_blockhelpmng(gcb));
      mask Display: disp('ATC.DSRVEC')
  SubSystem412 : Subsystem
      settings: RTWSystemCode=Inline
  Block936 : Outport
  Block937 : Outport  Port=2
  wires:
  Block925/1 -> Block936/1
  Block922/1 -> Block925/1
  Block921/1 -> Block926/1
  Block918/1 -> Block926/2
  SubSystem411/1(Output) -> SubSystem410/1(mult)
  Block915/1 -> SubSystem410/3(init)
  SubSystem410/1(Out) -> Block926/3  "SigLabel21"
  Block911/1 -> Block921/1
  SubSystem412/1(Block935) -> Block922/1, Block927/2, Block923/1
  Block913/1 -> Block926/4
  Block914/1 -> SubSystem412/1(Block928)
  Block926/1 -> SubSystem412/2(Block929)  "SigLabel20"
  Block916/1 -> SubSystem412/3(Block930)
  Block912/1 -> SubSystem411/1(element)
  Block917/1 -> Block923/2
  Block923/1 -> Block924/1
  Block924/1 -> Block920/1
  Block920/1 -> Block927/1
  Block927/1 -> Block937/1
  Block919/1 -> SubSystem410/2(div)

== system lib_all/SubSystem347/SubSystem349/SubSystem352/SubSystem406/SubSystem415 ==
  note: Text63
  note: Text62
  note: Text61
  Block938 : Inport
  Block939 : Inport  Port=2
  Block940 : Inport  Port=3
  Block941 : Constant  Value=0  OutDataTypeStr=double  SampleTime=-1
  Block942 : Constant  OutDataTypeStr=double  SampleTime=-1
  Block943 : Constant  OutDataTypeStr=double  SampleTime=-1
  Block944 : Constant  Value=0  OutDataTypeStr=double  SampleTime=-1
  Block945 : Constant  Value=2  OutDataTypeStr=double  SampleTime=-1
  Block946 : Constant  OutDataTypeStr=double  SampleTime=-1
  Block947 : Constant  Value=143  OutDataTypeStr=double  SampleTime=-1
  Block948 : Demux  Outputs=2
  Block949 : Logic  Operator=NOT
  Block950 : Mux  Inputs=2
  Block951 : Mux  Inputs=2
  Block952 : Sum  OutDataTypeStr=double  RndMeth=Zero  SaturateOnIntegerOverflow=on  InputSameDT=on
  Block953 : Sum  OutDataTypeStr=double  RndMeth=Zero  SaturateOnIntegerOverflow=on  InputSameDT=on
  Block{954..957} (4 alike) : Switch  Threshold=0.5  RndMeth=Zero  SaturateOnIntegerOverflow=on  InputSameDT=on
  SubSystem416 : Subsystem
  SubSystem417 : Subsystem
  SubSystem418 : Subsystem
  SubSystem419 : Subsystem
  SubSystem420 : Subsystem  TreatAsAtomicUnit=on
  SubSystem429 : Subsystem  TreatAsAtomicUnit=on
  SubSystem439 : Subsystem  TreatAsAtomicUnit=on
  SubSystem449 : Subsystem  TreatAsAtomicUnit=on
  Block1017 : Outport
  Block1018 : Outport  Port=2
  wires:
  Block956/1 -> Block953/2
  Block946/1 -> Block953/1
  Block938/1 -> SubSystem416/1(In1), Block952/2, Block950/1
  Block947/1 -> SubSystem416/2(In2), Block951/1
  Block939/1 -> SubSystem417/1(In1), SubSystem418/1(In1), SubSystem419/1(In1)
  Block941/1 -> SubSystem417/2(In2)
  SubSystem417/1(Out1) -> Block954/2, SubSystem420/Enable
  SubSystem420/1(Block971) -> Block954/1
  Block942/1 -> SubSystem418/2(In2)
  SubSystem418/1(Out1) -> Block955/2, SubSystem429/Enable
  SubSystem429/1(Block986) -> Block955/1
  Block955/1 -> Block954/3
  Block953/1 -> SubSystem420/1(Block958), SubSystem429/1(Block972), SubSystem439/1(Block987), SubSystem449/1(Block1002)
  Block948/1 -> Block1017/1
  Block948/2 -> Block1018/1
  Block954/1 -> Block948/1
  SubSystem416/1(Out1) -> Block956/2
  Block943/1 -> Block952/1
  Block944/1 -> Block951/2
  Block951/1 -> Block956/3
  Block952/1 -> Block950/2
  Block950/1 -> Block956/1
  Block945/1 -> SubSystem419/2(In2)
  SubSystem419/1(Out1) -> Block949/1, SubSystem439/Enable, Block957/2
  Block949/1 -> SubSystem449/Enable
  SubSystem439/1(Block1001) -> Block957/1
  SubSystem449/1(Block1016) -> Block957/3
  Block957/1 -> Block955/3
  Block940/1 -> SubSystem420/2(Block959), SubSystem429/2(Block973), SubSystem439/2(Block988), SubSystem449/2(Block1003)

== system lib_all/SubSystem347/SubSystem349/SubSystem352/SubSystem459/SubSystem460 ==
  Block1025 : Inport
  Block1026 : Inport  Port=2
  Block1027 : ActionPort
  SubSystem461 : Subsystem  TreatAsAtomicUnit=on  mask(***ATC.DSWVEC***){bd_version=1.01.A, r0=DSM382, r1=-1, r2=, r3=, r4=, r5=, r6=, r7=, r8=, r9=, c0=, c1=, c2=, c3=, c4=, c5=, c6=, c7=, c8=, c9=}
      mask prompts: bd_version "Version"
      mask help: これはAutoCからのブロックです．編集禁止．
      settings: OpenFcn=warndlg('変更禁止!');
      mask Help: web(ui_blockhelpmng(gcb));
      mask Display: disp('ATC.DSRVEC')
  wires:
  Block1025/1 -> SubSystem461/1(input)
  Block1026/1 -> SubSystem461/2(element)

== system lib_all/SubSystem347/SubSystem349/SubSystem352/SubSystem459/SubSystem462 ==
  Block1028 : Inport
  Block1029 : Inport  Port=2
  Block1030 : ActionPort
  SubSystem463 : Subsystem  TreatAsAtomicUnit=on  mask(***ATC.DSWVEC***){bd_version=1.01.A, r0=DSM391, r1=-1, r2=, r3=, r4=, r5=, r6=, r7=, r8=, r9=, c0=, c1=, c2=, c3=, c4=, c5=, c6=, c7=, c8=, c9=}
      mask prompts: bd_version "Version"
      mask help: これはAutoCからのブロックです．編集禁止．
      settings: OpenFcn=warndlg('変更禁止!');
      mask Help: web(ui_blockhelpmng(gcb));
      mask Display: disp('ATC.DSRVEC')
  wires:
  Block1028/1 -> SubSystem463/1(input)
  Block1029/1 -> SubSystem463/2(element)

== system lib_all/SubSystem347/SubSystem349/SubSystem352/SubSystem459/SubSystem464 ==
  Block1031 : Inport
  Block1032 : Inport  Port=2
  Block1033 : ActionPort
  SubSystem465 : Subsystem  TreatAsAtomicUnit=on  mask(***ATC.DSWVEC***){bd_version=1.01.A, r0=DSM393, r1=-1, r2=, r3=, r4=, r5=, r6=, r7=, r8=, r9=, c0=, c1=, c2=, c3=, c4=, c5=, c6=, c7=, c8=, c9=}
      mask prompts: bd_version "Version"
      mask help: これはAutoCからのブロックです．編集禁止．
      settings: OpenFcn=warndlg('変更禁止!');
      mask Help: web(ui_blockhelpmng(gcb));
      mask Display: disp('ATC.DSRVEC')
  wires:
  Block1031/1 -> SubSystem465/1(input)
  Block1032/1 -> SubSystem465/2(element)

== system lib_all/SubSystem347/SubSystem349/SubSystem352/SubSystem466/SubSystem467 ==
  Block1039 : Inport
  Block1040 : Inport  Port=2
  Block1041 : ActionPort
  SubSystem468 : Subsystem  TreatAsAtomicUnit=on  mask(***ATC.DSWVEC***){bd_version=1.01.A, r0=DSM384, r1=-1, r2=, r3=, r4=, r5=, r6=, r7=, r8=, r9=, c0=, c1=, c2=, c3=, c4=, c5=, c6=, c7=, c8=, c9=}
      mask prompts: bd_version "Version"
      mask help: これはAutoCからのブロックです．編集禁止．
      settings: OpenFcn=warndlg('変更禁止!');
      mask Help: web(ui_blockhelpmng(gcb));
      mask Display: disp('ATC.DSRVEC')
  wires:
  Block1040/1 -> SubSystem468/2(element)
  Block1039/1 -> SubSystem468/1(input)
